simulink model "Complete_Model"  (R2023a)
settings: Solver=VariableStepAuto  StartTime=0.0  StopTime=22  MinStep=5e-5  RelTol=1e-3
values from neighbouring files (symbols this model uses):
  Cbus = 80e-3*n_wt_agg*u^2    (= 1.33333333333)    [param.m]
  D = 1e-3    (= 0.001)    [param.m]
  f = 50    [param.m]
  Fs = Vs * sqrt(2/3)/(2*pi*f)    [param.m]
  fsw = 4e3    (= 4000)    [param.m]
  Idr_rated = sqrt(2)*Is    [param.m]
  J = 127*n_wt_agg    (= 19050)    [param.m]
  ki_id = (wni^2)*Lr*sigma    (= 3.27733001332)    [param.m]
  ki_idg = (wnig^2)*Lg    (= 0.263189450696)    [param.m]
  ki_iq = ki_id    (= 3.27733001332)    [param.m]
  ki_iqg = ki_idg    (= 0.263189450696)    [param.m]
  ki_v = -300000*n_wt_agg    (= -45000000)    [param.m]
  Kopt = ((0.5*rho*pi*(Radius^5)*Cp_max)/(lambda_opt^3))    (= 296454.198186)    [param.m]
  kp_id = (2*wni*sigma*Lr) - Rr    (= 0.00384733333333)    [param.m]
  kp_idg = ((2*wnig*Lg)-Rg)    (= 0.00167538274858)    [param.m]
  kp_iq = kp_id    (= 0.00384733333333)    [param.m]
  kp_iqg = kp_idg    (= 0.00167538274858)    [param.m]
  kp_v = -1000*n_wt_agg    (= -150000)    [param.m]
  Kpg = 1/(1.5*Vr*sqrt(2/3))    [param.m]
  Kqg = -Kpg    [param.m]
  lambda_opt = 7.2    [param.m]
  Lg = 400e-6 /n_wt_agg    (= 2.66666666667e-06)    [param.m]
  Lm = 2.5e-3 /n_wt_agg    (= 1.66666666667e-05)    [param.m]
  Lr = Lm + Lsi    (= 1.72466666667e-05)    [param.m]
  Ls = Lm + Lsi    (= 1.72466666667e-05)    [param.m]
  Lsi = 0.087e-3 /n_wt_agg    (= 5.8e-07)    [param.m]
  N = 100    [param.m]
  n_wt_agg = 150    [param.m]
  p = 2    [param.m]
  Ps = 2e6*n_wt_agg    (= 300000000)    [param.m]
  Radius = 42    [param.m]
  Rg = 20e-6 /n_wt_agg    (= 1.33333333333e-07)    [param.m]
  rho = 1.225    [param.m]
  Rr = 2.9e-3 /n_wt_agg    (= 1.93333333333e-05)    [param.m]
  Rs = 2.6e-3 /n_wt_agg    (= 1.73333333333e-05)    [param.m]
  sigma = 1 - Lm^2 / (Ls*Lr)    (= 0.0661284179513)    [param.m]
  smax = 1/3    (= 0.333333333333)    [param.m]
  tab_lambda = [0.1:0.01:11.8]    [param.m]
  Vbus = 1150/u    (= 3450)    [param.m]
  Vs = 690    [param.m]
undefined symbols (not defined in the file or in its folder): Cp, D1, Delta, DiscIntNeverNeededParam, magnitude
libraries: spsSecondOrderFilterLib x133, spsThreePhaseVIMeasurementLib x58, spsPower3phInstantaneousLib x52, simulink x41, spsThreePhaseTransformerTwoWindingsLib x27, spsUniversalBridgeLib x22, spsPWMGenerator2LevelLib x22, spsSeriesRLCBranchLib x19, spsFirstOrderFilterLib x18, spsThreePhasePISectionLineLib x17, spsGroundLib x17, spsThreePhaseSeriesRLCBranchLib x17
(part 1 of 15)
settings Model: Creator=deleersnijde  LastModifiedBy=deleersnijde
settings DataIOCC: ReturnWorkspaceOutputs=off
settings RTWCC: GPUComputeCapability=5.0

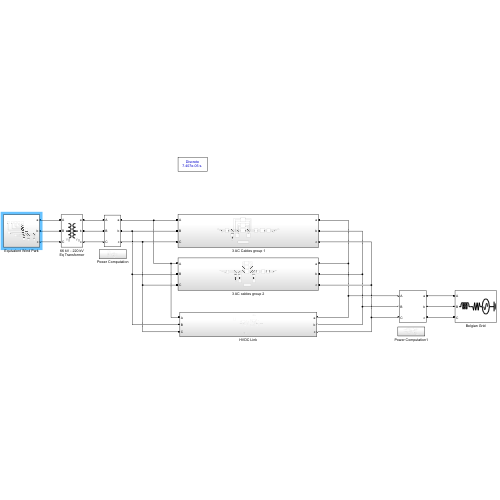
[thumbnail: Simulink's stored preview of the top-level diagram]
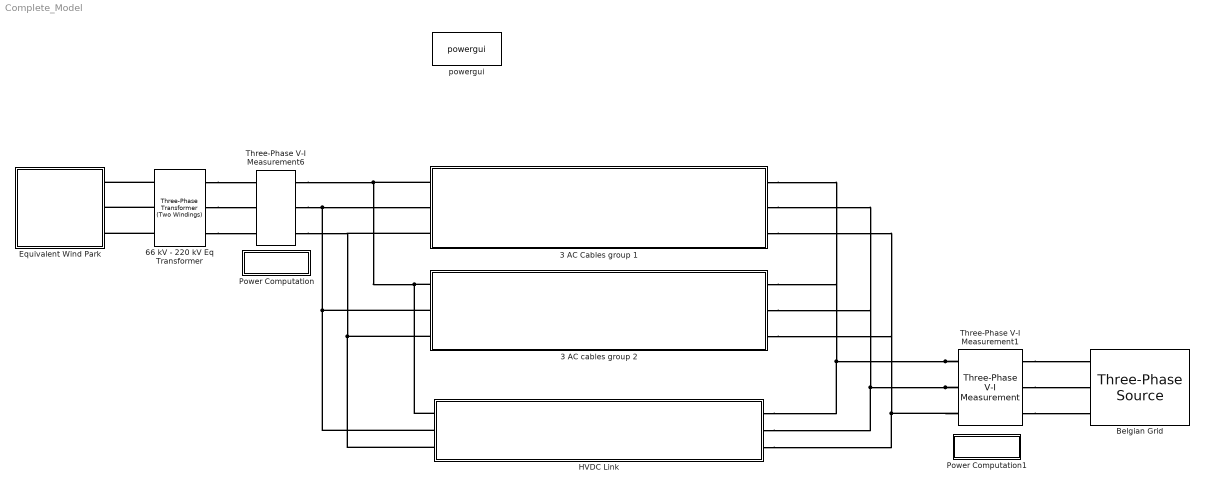
== system Complete_Model ==
  " 3 AC Cables group 1" : Subsystem
  3 AC cables group 2 : Subsystem
  66 kV - 220 kV Eq Transformer : spsThreePhaseTransformerTwoWindingsLib/Three-Phase Transformer (Two Windings)  Winding1Connection=Yg  Winding2Connection=Yg  CoreType=Three single-phase transformers  SetSaturation=off  Hysteresis=off  DataFile='hysteresis'  SetInitialFlux=off  Measurements=None  UNITS=pu  NominalPower=[ 3.5e9 , f ]  Winding1=[66e3 0.025/100 0.015]  Winding2=[220e3 0.025/100 0.015]  Rm=500  Lm=500  L0=0.5  Saturation=[0 0;0.22957 0.012546;95.654 0.015891]  InitialFluxes=[ 0.8 , -0.8 , 0.7 ]  BreakLoop=off  DiscreteSolver=Trapezoidal iterative  DataType=off  TransfoNumber=1
  Belgian Grid : spsThreePhaseSourceLib/Three-Phase Source  InternalConnection=Yg  VoltagePhases=off  Voltage=380e3  PhaseAngle=0  Voltage_phases=[25e3 25e3 25e3]/sqrt(3)  PhaseAngles_phases=[0 -120 +120]  Frequency=50  NonIdealSource=on  SpecifyImpedance=on  Resistance=0.8929  Inductance=16.58e-3  ShortCircuitLevel=1000000e6  BaseVoltage=380e3  XRratio=7  BusType=swing  Pref=10e3  Qref=0  Prefabc=[10e3, 10e3, 10e3]  Qrefabc=[0, 0, 0]  Qmin=-inf  Qmax=inf
  Equivalent Wind Park : Subsystem
  HVDC Link : Subsystem
  Power Computation : Subsystem
  Power Computation1 : Subsystem
  Three-Phase V-I Measurement1 : spsThreePhaseVIMeasurementLib/Three-Phase V-I Measurement  VoltageMeasurement=phase-to-ground  SetLabelV=on  LabelV=V_BE_grid_tot  Vpu=off  VpuLL=off  CurrentMeasurement=yes  SetLabelI=on  LabelI=I_BE_grid_tot  Ipu=off  Pbase=3*350e6  Vbase=66e3  OutputType=Complex  PhasorSimulation=off  PSBequivalent=0
  Three-Phase V-I Measurement6 : spsThreePhaseVIMeasurementLib/Three-Phase V-I Measurement  VoltageMeasurement=phase-to-ground  SetLabelV=on  LabelV=Vwf_tot  Vpu=off  VpuLL=off  CurrentMeasurement=yes  SetLabelI=on  LabelI=Iwf_tot  Ipu=off  Pbase=3*350e6  Vbase=66e3  OutputType=Complex  PhasorSimulation=off  PSBequivalent=0
  powergui : spspowerguiLib/powergui  Priority=1  SimulationMode=Discrete  SampleTime=7.4074e-05  frequency=60  Iterations=50  frequencyindice=50  Pbase=100e6  ErrMax=1e-4  UnitsV=kV  UnitsW=MW  buscounter=0  FunctionMessages=off  echomessages=off  EnableUseOfTLC=off  CurrentSourceSwitches=off  DisableSnubberDevices=off  DisableRonSwitches=off  DisableVfSwitches=off  DisplayEquations=off  AutomaticDiscreteSolvers=off  SolverType=Tustin/Backward Euler (TBE)  Interpol=off  ExternalGateDelay=off  methode=off  Ts=100  x0status=blocks  SPID=on  SwTol=0  HookPort=off  NonlinearTolerance=1e-4  nMaxIteration=100  ContinueOnMaxIteration=off  RestoreLinks=warning  ResistiveCurrentMeasurement=off  Frange=[0:2:500]  Ylog=off  Xlog=on  ShowGrid=off  save=off  variable=ZData  structure=0  ZoomFFT=off  StartTime=0.0  cycles=1  DisplayStyle=1  fundamental=60  FreqAxis=off  MaxFrequency=1000  frequencyindicesteady=1  RmsSteady=1  display=off
  connections:
  " 3 AC Cables group 1"/RConn3 -- 3 AC cables group 2/RConn3 -- HVDC Link/RConn3 -- Three-Phase V-I Measurement1/LConn3
  Three-Phase V-I Measurement1/LConn1 -- HVDC Link/RConn1 -- " 3 AC Cables group 1"/RConn1 -- 3 AC cables group 2/RConn1
  Three-Phase V-I Measurement1/LConn2 -- HVDC Link/RConn2 -- " 3 AC Cables group 1"/RConn2 -- 3 AC cables group 2/RConn2
  3 AC cables group 2/LConn2 -- HVDC Link/LConn2 -- " 3 AC Cables group 1"/LConn2 -- Three-Phase V-I Measurement6/RConn2
  3 AC cables group 2/LConn3 -- HVDC Link/LConn3 -- " 3 AC Cables group 1"/LConn3 -- Three-Phase V-I Measurement6/RConn3
  " 3 AC Cables group 1"/LConn1 -- Three-Phase V-I Measurement6/RConn1 -- HVDC Link/LConn1 -- 3 AC cables group 2/LConn1
  Three-Phase V-I Measurement1/RConn1 -- Belgian Grid/RConn1
  Three-Phase V-I Measurement1/RConn3 -- Belgian Grid/RConn3
  Three-Phase V-I Measurement1/RConn2 -- Belgian Grid/RConn2
  66 kV - 220 kV Eq Transformer/RConn1 -- Three-Phase V-I Measurement6/LConn1
  66 kV - 220 kV Eq Transformer/RConn2 -- Three-Phase V-I Measurement6/LConn2
  66 kV - 220 kV Eq Transformer/RConn3 -- Three-Phase V-I Measurement6/LConn3
  66 kV - 220 kV Eq Transformer/LConn1 -- Equivalent Wind Park/RConn1
  66 kV - 220 kV Eq Transformer/LConn2 -- Equivalent Wind Park/RConn2
  66 kV - 220 kV Eq Transformer/LConn3 -- Equivalent Wind Park/RConn3

== system Complete_Model/ 3 AC Cables group 1 ==
  220kV - 380kV : spsThreePhaseTransformerTwoWindingsLib/Three-Phase Transformer (Two Windings)  Winding1Connection=Yg  Winding2Connection=Yg  CoreType=Three single-phase transformers  SetSaturation=off  Hysteresis=off  DataFile='hysteresis'  SetInitialFlux=off  Measurements=None  UNITS=pu  NominalPower=[ 3*350e6 , 50 ]  Winding1=[ 220e3 , 0.002 , 0.08 ]  Winding2=[ 380e3 , 0.002 , 0.08 ]  Rm=500  Lm=500  L0=0.5  Saturation=[ 0,0 ; 0.0024,1.2 ; 1.0,1.52 ]  InitialFluxes=[ 0.8 , -0.8 , 0.7 ]  BreakLoop=off  DiscreteSolver=Trapezoidal iterative  DataType=off  TransfoNumber=1
  220kV export cable1 : spsThreePhasePISectionLineLib/Three-Phase PI Section Line  LConnTagsString=a|b|c  RConnTagsString=A|B|C  Length=45  Frequency=50  Resistances=[ 0.048 0.048]  Inductances="[ 0.37e-3  0.37e-3]"  Capacitances=[0.18e-6 0.18e-6]
  220kV export cable2 : spsThreePhasePISectionLineLib/Three-Phase PI Section Line  LConnTagsString=a|b|c  RConnTagsString=A|B|C  Length=45  Frequency=50  Resistances=[ 0.048 0.048]  Inductances="[ 0.37e-3  0.37e-3]"  Capacitances=[0.18e-6 0.18e-6]
  220kV export cable3 : spsThreePhasePISectionLineLib/Three-Phase PI Section Line  LConnTagsString=a|b|c  RConnTagsString=A|B|C  Length=45  Frequency=50  Resistances=[ 0.048 0.048]  Inductances="[ 0.37e-3  0.37e-3]"  Capacitances=[0.18e-6 0.18e-6]
  Ground11 : spsGroundLib/Ground  LConnTagsString=a
  Power Computations : Subsystem
  Shunt Reactor 1 : spsThreePhaseSeriesRLCLoadLib/Three-Phase Series RLC Load  LConnTagsString=A|B|C  Configuration=Y (grounded)  NominalVoltage=220e3  Vabc=[1e3 1e3 1e3]  Vabcp=[1e3 1e3 1e3]  NominalFrequency=50  UnbalancedPower=off  ActivePower=0  InductivePower=175e6  CapacitivePower=0  Pabc=[10e3 9e3 11e3]  QLabc=[100 90 110]  QCabc=[100 90 110]  Pabcp=[10e3 9e3 11e3]  QLabcp=[100 90 110]  QCabcp=[100 90 110]  Measurements=None  LoadType=constant Z
  Shunt Reactor 2 : spsThreePhaseSeriesRLCLoadLib/Three-Phase Series RLC Load  LConnTagsString=A|B|C  Configuration=Y (grounded)  NominalVoltage=220e3  Vabc=[1e3 1e3 1e3]  Vabcp=[1e3 1e3 1e3]  NominalFrequency=50  UnbalancedPower=off  ActivePower=0  InductivePower=175e6  CapacitivePower=0  Pabc=[10e3 9e3 11e3]  QLabc=[100 90 110]  QCabc=[100 90 110]  Pabcp=[10e3 9e3 11e3]  QLabcp=[100 90 110]  QCabcp=[100 90 110]  Measurements=None  LoadType=constant Z
  Snubber Shunt5 : spsThreePhaseSeriesRLCBranchLib/Three-Phase Series RLC Branch  LConnTagsString=A|B|C  RConnTagsString=A|B|C  BranchType=R  Resistance=10e6  Inductance=Lg  Capacitance=1e-6  Measurements=None
  Three-Phase V-I Measurement3 : spsThreePhaseVIMeasurementLib/Three-Phase V-I Measurement  VoltageMeasurement=phase-to-ground  SetLabelV=on  LabelV=Vcontinent220  Vpu=off  VpuLL=off  CurrentMeasurement=yes  SetLabelI=on  LabelI=Icontinent220  Ipu=off  Pbase=3*350e6  Vbase=220e3  OutputType=Complex  PhasorSimulation=off  PSBequivalent=0
  Three-Phase V-I Measurement6 : spsThreePhaseVIMeasurementLib/Three-Phase V-I Measurement  VoltageMeasurement=phase-to-ground  SetLabelV=on  LabelV=Ven_isl  Vpu=off  VpuLL=off  CurrentMeasurement=yes  SetLabelI=on  LabelI=Ien_isl  Ipu=off  Pbase=3*350e6  Vbase=66e3  OutputType=Complex  PhasorSimulation=off  PSBequivalent=0
  Three-Phase V-I Measurement8 : spsThreePhaseVIMeasurementLib/Three-Phase V-I Measurement  VoltageMeasurement=phase-to-ground  SetLabelV=on  LabelV=Vcontinent  Vpu=off  VpuLL=off  CurrentMeasurement=yes  SetLabelI=on  LabelI=Icontinent  Ipu=off  Pbase=3*350e6  Vbase=380e3  OutputType=Complex  PhasorSimulation=off  PSBequivalent=0
  a : PMIOPort  Side=Right
  b : PMIOPort  Port=2  Side=Right
  c : PMIOPort  Port=3  Side=Right
  A : PMIOPort  Port=4  Side=Left
  B : PMIOPort  Port=5  Side=Left
  C : PMIOPort  Port=6  Side=Left
  connections:
  Three-Phase V-I Measurement6/RConn2 -- 220kV export cable2/LConn2 -- 220kV export cable3/LConn2 -- Shunt Reactor 1/LConn2 -- 220kV export cable1/LConn2 -- Snubber Shunt5/LConn2
  Shunt Reactor 2/LConn3 -- Three-Phase V-I Measurement3/LConn3 -- 220kV export cable2/RConn3 -- 220kV export cable3/RConn3 -- 220kV export cable1/RConn3
  Snubber Shunt5/RConn2 -- Snubber Shunt5/RConn3 -- Ground11/LConn1 -- Snubber Shunt5/RConn1
  Shunt Reactor 2/LConn1 -- 220kV export cable3/RConn1 -- 220kV export cable1/RConn1 -- Three-Phase V-I Measurement3/LConn1 -- 220kV export cable2/RConn1
  Three-Phase V-I Measurement8/LConn2 -- 220kV - 380kV/RConn2
  Snubber Shunt5/LConn3 -- Shunt Reactor 1/LConn3 -- 220kV export cable2/LConn3 -- 220kV export cable3/LConn3 -- 220kV export cable1/LConn3 -- Three-Phase V-I Measurement6/RConn3
  Shunt Reactor 2/LConn2 -- 220kV export cable2/RConn2 -- 220kV export cable3/RConn2 -- Three-Phase V-I Measurement3/LConn2 -- 220kV export cable1/RConn2
  Three-Phase V-I Measurement3/RConn1 -- 220kV - 380kV/LConn1
  Three-Phase V-I Measurement6/RConn1 -- Shunt Reactor 1/LConn1 -- 220kV export cable2/LConn1 -- 220kV export cable3/LConn1 -- 220kV export cable1/LConn1 -- Snubber Shunt5/LConn1
  Three-Phase V-I Measurement3/RConn2 -- 220kV - 380kV/LConn2
  Three-Phase V-I Measurement8/LConn1 -- 220kV - 380kV/RConn1
  Three-Phase V-I Measurement3/RConn3 -- 220kV - 380kV/LConn3
  Three-Phase V-I Measurement8/LConn3 -- 220kV - 380kV/RConn3
  Three-Phase V-I Measurement8/RConn1 -- a/RConn1
  Three-Phase V-I Measurement8/RConn3 -- c/RConn1
  Three-Phase V-I Measurement8/RConn2 -- b/RConn1
  B/RConn1 -- Three-Phase V-I Measurement6/LConn2
  C/RConn1 -- Three-Phase V-I Measurement6/LConn3
  A/RConn1 -- Three-Phase V-I Measurement6/LConn1

== system Complete_Model/3 AC cables group 2 ==
  220kV - 380kV1 : spsThreePhaseTransformerTwoWindingsLib/Three-Phase Transformer (Two Windings)  Winding1Connection=Yg  Winding2Connection=Yg  CoreType=Three single-phase transformers  SetSaturation=off  Hysteresis=off  DataFile='hysteresis'  SetInitialFlux=off  Measurements=None  UNITS=pu  NominalPower=[ 3*350e6 , 50 ]  Winding1=[ 220e3 , 0.002 , 0.08 ]  Winding2=[ 380e3 , 0.002 , 0.08 ]  Rm=500  Lm=500  L0=0.5  Saturation=[ 0,0 ; 0.0024,1.2 ; 1.0,1.52 ]  InitialFluxes=[ 0.8 , -0.8 , 0.7 ]  BreakLoop=off  DiscreteSolver=Trapezoidal iterative  DataType=off  TransfoNumber=1
  220kV export cable4 : spsThreePhasePISectionLineLib/Three-Phase PI Section Line  LConnTagsString=a|b|c  RConnTagsString=A|B|C  Length=45  Frequency=50  Resistances=[ 0.048 0.048]  Inductances="[ 0.37e-3  0.37e-3]"  Capacitances=[0.18e-6 0.18e-6]
  220kV export cable5 : spsThreePhasePISectionLineLib/Three-Phase PI Section Line  LConnTagsString=a|b|c  RConnTagsString=A|B|C  Length=45  Frequency=50  Resistances=[ 0.048 0.048]  Inductances="[ 0.37e-3  0.37e-3]"  Capacitances=[0.18e-6 0.18e-6]
  220kV export cable6 : spsThreePhasePISectionLineLib/Three-Phase PI Section Line  LConnTagsString=a|b|c  RConnTagsString=A|B|C  Length=45  Frequency=50  Resistances=[ 0.048 0.048]  Inductances="[ 0.37e-3  0.37e-3]"  Capacitances=[0.18e-6 0.18e-6]
  Ground3 : spsGroundLib/Ground  LConnTagsString=a
  Power Computations : Subsystem
  Snubber Shunt4 : spsThreePhaseSeriesRLCBranchLib/Three-Phase Series RLC Branch  LConnTagsString=A|B|C  RConnTagsString=A|B|C  BranchType=R  Resistance=10e6  Inductance=Lg  Capacitance=1e-6  Measurements=None
  Three-Phase Series RLC Load2 : spsThreePhaseSeriesRLCLoadLib/Three-Phase Series RLC Load  LConnTagsString=A|B|C  Configuration=Y (grounded)  NominalVoltage=220e3  Vabc=[1e3 1e3 1e3]  Vabcp=[1e3 1e3 1e3]  NominalFrequency=50  UnbalancedPower=off  ActivePower=0  InductivePower=175e6  CapacitivePower=0  Pabc=[10e3 9e3 11e3]  QLabc=[100 90 110]  QCabc=[100 90 110]  Pabcp=[10e3 9e3 11e3]  QLabcp=[100 90 110]  QCabcp=[100 90 110]  Measurements=None  LoadType=constant Z
  Three-Phase Series RLC Load3 : spsThreePhaseSeriesRLCLoadLib/Three-Phase Series RLC Load  LConnTagsString=A|B|C  Configuration=Y (grounded)  NominalVoltage=220e3  Vabc=[1e3 1e3 1e3]  Vabcp=[1e3 1e3 1e3]  NominalFrequency=50  UnbalancedPower=off  ActivePower=0  InductivePower=175e6  CapacitivePower=0  Pabc=[10e3 9e3 11e3]  QLabc=[100 90 110]  QCabc=[100 90 110]  Pabcp=[10e3 9e3 11e3]  QLabcp=[100 90 110]  QCabcp=[100 90 110]  Measurements=None  LoadType=constant Z
  Three-Phase V-I Measurement1 : spsThreePhaseVIMeasurementLib/Three-Phase V-I Measurement  VoltageMeasurement=phase-to-ground  SetLabelV=on  LabelV=Vsh_wind2  Vpu=off  VpuLL=off  CurrentMeasurement=yes  SetLabelI=on  LabelI=Ish_wind2  Ipu=off  Pbase=3*350e6  Vbase=66e3  OutputType=Complex  PhasorSimulation=off  PSBequivalent=0
  Three-Phase V-I Measurement10 : spsThreePhaseVIMeasurementLib/Three-Phase V-I Measurement  VoltageMeasurement=phase-to-ground  SetLabelV=on  LabelV=Ven_isl2  Vpu=off  VpuLL=off  CurrentMeasurement=yes  SetLabelI=on  LabelI=Ien_isl2  Ipu=off  Pbase=3*350e6  Vbase=66e3  OutputType=Complex  PhasorSimulation=off  PSBequivalent=0
  Three-Phase V-I Measurement11 : spsThreePhaseVIMeasurementLib/Three-Phase V-I Measurement  VoltageMeasurement=phase-to-ground  SetLabelV=on  LabelV=Vcontinent2  Vpu=off  VpuLL=off  CurrentMeasurement=yes  SetLabelI=on  LabelI=Icontinent2  Ipu=off  Pbase=3*350e6  Vbase=380e3  OutputType=Complex  PhasorSimulation=off  PSBequivalent=0
  Three-Phase V-I Measurement2 : spsThreePhaseVIMeasurementLib/Three-Phase V-I Measurement  VoltageMeasurement=phase-to-ground  SetLabelV=on  LabelV=Vsh_grid2  Vpu=off  VpuLL=off  CurrentMeasurement=yes  SetLabelI=on  LabelI=Ish_grid2  Ipu=off  Pbase=3*350e6  Vbase=66e3  OutputType=Complex  PhasorSimulation=off  PSBequivalent=0
  Three-Phase V-I Measurement9 : spsThreePhaseVIMeasurementLib/Three-Phase V-I Measurement  VoltageMeasurement=phase-to-ground  SetLabelV=on  LabelV=Vcontinent220_2  Vpu=off  VpuLL=off  CurrentMeasurement=yes  SetLabelI=on  LabelI=Icontinent220_2  Ipu=off  Pbase=3*350e6  Vbase=220e3  OutputType=Complex  PhasorSimulation=off  PSBequivalent=0
  a : PMIOPort  Side=Right
  b : PMIOPort  Port=2  Side=Right
  c : PMIOPort  Port=3  Side=Right
  A : PMIOPort  Port=4  Side=Left
  B : PMIOPort  Port=5  Side=Left
  C : PMIOPort  Port=6  Side=Left
  connections:
  Three-Phase V-I Measurement9/RConn2 -- 220kV - 380kV1/LConn2
  Three-Phase V-I Measurement9/LConn2 -- 220kV export cable6/RConn2 -- 220kV export cable4/RConn2 -- 220kV export cable5/RConn2 -- Three-Phase V-I Measurement2/LConn2
  220kV export cable6/RConn1 -- Three-Phase V-I Measurement2/LConn1 -- Three-Phase V-I Measurement9/LConn1 -- 220kV export cable4/RConn1 -- 220kV export cable5/RConn1
  220kV export cable6/RConn3 -- Three-Phase V-I Measurement2/LConn3 -- Three-Phase V-I Measurement9/LConn3 -- 220kV export cable5/RConn3 -- 220kV export cable4/RConn3
  Three-Phase V-I Measurement11/LConn2 -- 220kV - 380kV1/RConn2
  Three-Phase V-I Measurement11/RConn3 -- c/RConn1
  Three-Phase V-I Measurement10/LConn1 -- A/RConn1
  Three-Phase V-I Measurement10/RConn3 -- Snubber Shunt4/LConn3 -- Three-Phase V-I Measurement1/LConn3 -- 220kV export cable6/LConn3 -- 220kV export cable5/LConn3 -- 220kV export cable4/LConn3
  Three-Phase V-I Measurement11/LConn3 -- 220kV - 380kV1/RConn3
  220kV export cable6/LConn2 -- Three-Phase V-I Measurement1/LConn2 -- Three-Phase V-I Measurement10/RConn2 -- Snubber Shunt4/LConn2 -- 220kV export cable5/LConn2 -- 220kV export cable4/LConn2
  220kV export cable6/LConn1 -- Three-Phase V-I Measurement1/LConn1 -- Three-Phase V-I Measurement10/RConn1 -- Snubber Shunt4/LConn1 -- 220kV export cable4/LConn1 -- 220kV export cable5/LConn1
  Ground3/LConn1 -- Snubber Shunt4/RConn1 -- Snubber Shunt4/RConn3 -- Snubber Shunt4/RConn2
  Three-Phase V-I Measurement11/RConn2 -- b/RConn1
  Three-Phase V-I Measurement9/RConn3 -- 220kV - 380kV1/LConn3
  Three-Phase V-I Measurement10/LConn2 -- B/RConn1
  Three-Phase V-I Measurement11/LConn1 -- 220kV - 380kV1/RConn1
  Three-Phase V-I Measurement9/RConn1 -- 220kV - 380kV1/LConn1
  Three-Phase V-I Measurement11/RConn1 -- a/RConn1
  Three-Phase V-I Measurement10/LConn3 -- C/RConn1
  Three-Phase Series RLC Load2/LConn3 -- Three-Phase V-I Measurement1/RConn3
  Three-Phase Series RLC Load2/LConn2 -- Three-Phase V-I Measurement1/RConn2
  Three-Phase Series RLC Load2/LConn1 -- Three-Phase V-I Measurement1/RConn1
  Three-Phase V-I Measurement2/RConn1 -- Three-Phase Series RLC Load3/LConn1
  Three-Phase V-I Measurement2/RConn3 -- Three-Phase Series RLC Load3/LConn3
  Three-Phase Series RLC Load3/LConn2 -- Three-Phase V-I Measurement2/RConn2

== system Complete_Model/Equivalent Wind Park ==
  300MW Eq WT1 : Subsystem
  300MW Eq WT10 : Subsystem
  300MW Eq WT11 : Subsystem
  300MW Eq WT2 : Subsystem
  300MW Eq WT3 : Subsystem
  300MW Eq WT4 : Subsystem
  300MW Eq WT5 : Subsystem
  300MW Eq WT6 : Subsystem
  300MW Eq WT7 : Subsystem
  300MW Eq WT8 : Subsystem
  300MW Eq WT9 : Subsystem
  a : PMIOPort  Side=Right
  b : PMIOPort  Port=2  Side=Right
  c : PMIOPort  Port=3  Side=Right
  connections:
  300MW Eq WT1/LConn3 -- 300MW Eq WT3/LConn3 -- 300MW Eq WT4/LConn3 -- 300MW Eq WT5/LConn3 -- 300MW Eq WT6/LConn3 -- c/RConn1 -- 300MW Eq WT11/LConn3 -- 300MW Eq WT10/LConn3 -- 300MW Eq WT8/LConn3 -- 300MW Eq WT9/LConn3 -- 300MW Eq WT7/LConn3 -- 300MW Eq WT2/LConn3
  300MW Eq WT1/LConn1 -- 300MW Eq WT3/LConn1 -- 300MW Eq WT4/LConn1 -- 300MW Eq WT5/LConn1 -- 300MW Eq WT6/LConn1 -- 300MW Eq WT9/LConn1 -- 300MW Eq WT8/LConn1 -- a/RConn1 -- 300MW Eq WT11/LConn1 -- 300MW Eq WT10/LConn1 -- 300MW Eq WT7/LConn1 -- 300MW Eq WT2/LConn1
  300MW Eq WT1/LConn2 -- 300MW Eq WT3/LConn2 -- 300MW Eq WT4/LConn2 -- 300MW Eq WT5/LConn2 -- 300MW Eq WT6/LConn2 -- b/RConn1 -- 300MW Eq WT11/LConn2 -- 300MW Eq WT10/LConn2 -- 300MW Eq WT8/LConn2 -- 300MW Eq WT9/LConn2 -- 300MW Eq WT7/LConn2 -- 300MW Eq WT2/LConn2

== system Complete_Model/HVDC Link ==
  220 kV - 525 kV L = 0.1947 pu : spsThreePhaseTransformerTwoWindingsLib/Three-Phase Transformer (Two Windings)  Winding1Connection=Yg  Winding2Connection=Yg  CoreType=Three single-phase transformers  SetSaturation=off  Hysteresis=off  DataFile='hysteresis'  SetInitialFlux=off  Measurements=None  UNITS=pu  NominalPower=[ 2e9 , 50 ]  Winding1=[2.2e+05 0.0025 0.1947/2]  Winding2=[525e3 0.0025 0.1947/2]  Rm=500  Lm=500  L0=0.5  Saturation=[0 0;0.0003 1.2;0.125 1.52]  InitialFluxes=[ 0.8 , -0.8 , 0.7 ]  BreakLoop=off  DiscreteSolver=Trapezoidal iterative  DataType=off  TransfoNumber=1
  45-km cable neg dc : spsPiSectionLineLib/Pi Section Line  LConnTagsString=__new0  RConnTagsString=__new0  Phases=1  PiSections=1  Length=45  Frequency=50  Resistance=0.0072  Inductance=1.5e-004  Capacitance=0.22e-06  SpecifyConductance=off  Conductance=0  Measurements=None  Measurements2=None
  45-km cable pos dc : spsPiSectionLineLib/Pi Section Line  LConnTagsString=__new0  RConnTagsString=__new0  Phases=1  PiSections=1  Length=45  Frequency=50  Resistance=0.0072  Inductance=1.5e-004  Capacitance=0.22e-06  SpecifyConductance=off  Conductance=0  Measurements=None  Measurements2=None
  525 kV - 380 kV L = 0.1947 pu : spsThreePhaseTransformerTwoWindingsLib/Three-Phase Transformer (Two Windings)  Winding1Connection=Yg  Winding2Connection=Yg  CoreType=Three single-phase transformers  SetSaturation=off  Hysteresis=off  DataFile='hysteresis'  SetInitialFlux=off  Measurements=None  UNITS=pu  NominalPower=[ 2e9 , 50 ]  Winding1=[380e3 0.0025 0.1947/2]  Winding2=[525e3 0.0025 0.1947/2]  Rm=500  Lm=500  L0=0.5  Saturation=[0 0;0.0003 1.2;0.125 1.52]  InitialFluxes=[ 0.8 , -0.8 , 0.7 ]  BreakLoop=off  DiscreteSolver=Trapezoidal iterative  DataType=off  TransfoNumber=1
  AC Filter gride side : spsThreePhaseSeriesRLCBranchLib/Three-Phase Series RLC Branch  LConnTagsString=A|B|C  RConnTagsString=A|B|C  BranchType=RC  Resistance=62.64  Inductance=2.9042e-3  Capacitance=1.1549e-6  Measurements=None
  AC Filter wfside : spsThreePhaseSeriesRLCBranchLib/Three-Phase Series RLC Branch  LConnTagsString=A|B|C  RConnTagsString=A|B|C  BranchType=RC  Resistance=62.64  Inductance=2.9042e-3  Capacitance=1.1549e-6  Measurements=None
  Block2 : Step  Time=0.1  Before=1  After=0  SampleTime=7.4074e-05
  Block3 : Step  Time=0.3  Before=1  After=0  SampleTime=7.4074e-05
  Constant1 : Constant
  Constant10 : Constant
  Constant11 : Constant  Value=0
  Constant12 : Constant  Value=0
  Current Measurement1 : spsCurrentMeasurementLib/Current Measurement  PhasorSimulation=off  OutputType=Complex  PSBequivalent=0
  Current Measurement2 : spsCurrentMeasurementLib/Current Measurement  PhasorSimulation=off  OutputType=Complex  PSBequivalent=0
  DC Capacitor : spsSeriesRLCBranchLib/Series RLC Branch  LConnTagsString=__new0  RConnTagsString=__new0  BranchType=C  Resistance=1  Inductance=1e-3  SetiL0=off  InitialCurrent=0  Capacitance=25.4e-6  Setx0=off  InitialVoltage=525e5  Measurements=None
  Demux1 : Demux  Outputs=3
  Grid side control : Subsystem
  Ground{1..2,4,6} (4 alike) : spsGroundLib/Ground  LConnTagsString=a
  Lp2 : spsSeriesRLCBranchLib/Series RLC Branch  LConnTagsString=__new0  RConnTagsString=__new0  BranchType=RL  Resistance=0.0692  Inductance=0.02205  SetiL0=off  InitialCurrent=0  Capacitance=[]  Setx0=off  InitialVoltage=0  Measurements=Branch current
  Lp3 : spsSeriesRLCBranchLib/Series RLC Branch  LConnTagsString=__new0  RConnTagsString=__new0  BranchType=RL  Resistance=0.0692  Inductance=0.02205  SetiL0=off  InitialCurrent=0  Capacitance=[]  Setx0=off  InitialVoltage=0  Measurements=Branch current
  Lp4 : spsSeriesRLCBranchLib/Series RLC Branch  LConnTagsString=__new0  RConnTagsString=__new0  BranchType=RL  Resistance=0.0692  Inductance=0.02205  SetiL0=off  InitialCurrent=0  Capacitance=[]  Setx0=off  InitialVoltage=0  Measurements=Branch current
  PWM Generator (3-Level)2 : spsPWMGenerator3LevelLib/PWM Generator (3-Level)  ModulatorType=Three-phase bridge (12 pulses)  ModulatorMode=Unsynchronized  nF=27  Fc=27*50  ModulatingSignals=off  m=0.8  Freq=60  Phase=0  Ts=7.4074e-05
  PWM Generator (3-Level)3 : spsPWMGenerator3LevelLib/PWM Generator (3-Level)  ModulatorType=Three-phase bridge (12 pulses)  ModulatorMode=Unsynchronized  nF=27  Fc=27*50  ModulatingSignals=off  m=0.8  Freq=60  Phase=0  Ts=7.4074e-05
  Phase Reactor grid L = 0.0781 pu : spsThreePhaseSeriesRLCBranchLib/Three-Phase Series RLC Branch  LConnTagsString=A|B|C  RConnTagsString=A|B|C  BranchType=RL  Resistance=(0.015/100)*(525^2 / 2000)  Inductance=0.0781  Capacitance=1e-6  Measurements=None
  Phase Reactor wfside L = 0.0781 pu : spsThreePhaseSeriesRLCBranchLib/Three-Phase Series RLC Branch  LConnTagsString=A|B|C  RConnTagsString=A|B|C  BranchType=RL  Resistance=(0.015/100)*(525^2 / 2000)  Inductance=0.0781  Capacitance=1e-6  Measurements=None
  Power compuations Grid Side : Subsystem
  Power compuations Wind Farm Side : Subsystem
  Power losses & DC power : Subsystem
  Series RLC Branch4 : spsSeriesRLCBranchLib/Series RLC Branch  LConnTagsString=__new0  RConnTagsString=__new0  BranchType=C  Resistance=1  Inductance=1e-3  SetiL0=off  InitialCurrent=0  Capacitance=25.4e-6  Setx0=off  InitialVoltage=525e5  Measurements=None
  Series RLC Branch5 : spsSeriesRLCBranchLib/Series RLC Branch  LConnTagsString=__new0  RConnTagsString=__new0  BranchType=C  Resistance=1  Inductance=1e-3  SetiL0=off  InitialCurrent=0  Capacitance=25.4e-6  Setx0=off  InitialVoltage=525e5  Measurements=None
  Series RLC Branch6 : spsSeriesRLCBranchLib/Series RLC Branch  LConnTagsString=__new0  RConnTagsString=__new0  BranchType=C  Resistance=1  Inductance=1e-3  SetiL0=off  InitialCurrent=0  Capacitance=25.4e-6  Setx0=off  InitialVoltage=525e5  Measurements=None
  Smoothing Reactor : spsSeriesRLCBranchLib/Series RLC Branch  LConnTagsString=__new0  RConnTagsString=__new0  BranchType=RL  Resistance=0.0692  Inductance=0.02205  SetiL0=off  InitialCurrent=0  Capacitance=[]  Setx0=off  InitialVoltage=0  Measurements=Branch current
  Step3 : Step  Time=0.05  Before=1  After=-1  SampleTime=0
  Subsystem : Subsystem
  Subsystem1 : Subsystem
  Subsystem7 : Subsystem
  Switch3 : Switch  Criteria=u2 > Threshold  InputSameDT=off
  Switch4 : Switch  Criteria=u2 > Threshold  InputSameDT=off
  Switch5 : Switch  Criteria=u2 > Threshold  SaturateOnIntegerOverflow=off  InputSameDT=off
  Three-Level Bridge2 : spsThreeLevelBridgeLib/Three-Level Bridge  Arms=3  SnubberResistance=5000  SbubberCapacitance=1e-6  Device=IGBT / Diodes  Ron=1e-3  ForwardVoltages="[  0  0  ]"  Measurements=None
      settings: LibrarySourceBlock=sps_lib/Power Electronics/Three-Level Bridge
  Three-Level Bridge3 : spsThreeLevelBridgeLib/Three-Level Bridge  Arms=3  SnubberResistance=5000  SbubberCapacitance=1e-6  Device=IGBT / Diodes  Ron=1e-3  ForwardVoltages="[  0  0  ]"  Measurements=None
      settings: LibrarySourceBlock=sps_lib/Power Electronics/Three-Level Bridge
  Three-Phase V-I Measurement1 : spsThreePhaseVIMeasurementLib/Three-Phase V-I Measurement  VoltageMeasurement=phase-to-ground  SetLabelV=on  LabelV=Eabc_windfarm  Vpu=on  VpuLL=off  CurrentMeasurement=yes  SetLabelI=on  LabelI=Iabc_windfarm  Ipu=on  Pbase=2e9  Vbase=220e3  OutputType=Complex  PhasorSimulation=off  PSBequivalent=0
  Three-Phase V-I Measurement4 : spsThreePhaseVIMeasurementLib/Three-Phase V-I Measurement  VoltageMeasurement=phase-to-ground  SetLabelV=on  LabelV=Eabc_grid  Vpu=on  VpuLL=off  CurrentMeasurement=yes  SetLabelI=on  LabelI=Iabc_grid  Ipu=on  Pbase=2e9  Vbase=380e3  OutputType=Complex  PhasorSimulation=off  PSBequivalent=0
  Three-Phase V-I Measurement5 : spsThreePhaseVIMeasurementLib/Three-Phase V-I Measurement  VoltageMeasurement=phase-to-ground  SetLabelV=on  LabelV=Uconv_grid  Vpu=on  VpuLL=off  CurrentMeasurement=no  SetLabelI=on  LabelI=Iabc_grid  Ipu=on  Pbase=2e9  Vbase=525e3  OutputType=Complex  PhasorSimulation=off  PSBequivalent=0
  Three-Phase V-I Measurement7 : spsThreePhaseVIMeasurementLib/Three-Phase V-I Measurement  VoltageMeasurement=phase-to-ground  SetLabelV=on  LabelV=Uconv_windfarm  Vpu=on  VpuLL=off  CurrentMeasurement=yes  SetLabelI=on  LabelI=Iconv_windfarm  Ipu=on  Pbase=2e9  Vbase=525e3  OutputType=Complex  PhasorSimulation=off  PSBequivalent=0
  To Workspace1 : ToWorkspace  VariableName=simout1  SaveFormat=Structure With Time  SampleTime=-1
  To Workspace3 : ToWorkspace  VariableName=Vdc_ref  SaveFormat=Timeseries  SampleTime=-1
  ToFile_Vdc_ref : ToFile  Filename=Vdc_ref.mat  SaveFormat=Timeseries
  ToFile_simout1 : ToFile  Filename=simout1.mat  SaveFormat=Timeseries
  V_DC Measure : Subsystem
  V_DC_neg Measure : Subsystem
  Voltage Measurement5 : spsVoltageMeasurementLib/Voltage Measurement  PhasorSimulation=off  OutputType=Complex  PSBequivalent=0
  Wind farm side control : Subsystem
  big scope : Subsystem
  powergui : spspowerguiLib/powergui  Priority=1  SimulationMode=Discrete  SampleTime=7.4074e-05  frequency=60  Iterations=50  frequencyindice=50  Pbase=100e6  ErrMax=1e-4  UnitsV=kV  UnitsW=MW  buscounter=0  FunctionMessages=off  echomessages=off  EnableUseOfTLC=off  CurrentSourceSwitches=off  DisableSnubberDevices=off  DisableRonSwitches=off  DisableVfSwitches=off  DisplayEquations=off  AutomaticDiscreteSolvers=off  SolverType=Tustin/Backward Euler (TBE)  Interpol=off  ExternalGateDelay=off  methode=off  Ts=100  x0status=blocks  SPID=on  SwTol=0  HookPort=off  NonlinearTolerance=1e-4  nMaxIteration=100  ContinueOnMaxIteration=off  RestoreLinks=warning  ResistiveCurrentMeasurement=off  Frange=[0:2:500]  Ylog=off  Xlog=on  ShowGrid=off  save=off  variable=ZData  structure=0  ZoomFFT=off  StartTime=0.0  cycles=1  DisplayStyle=1  fundamental=60  FreqAxis=off  MaxFrequency=1000  frequencyindicesteady=1  RmsSteady=1  display=off
  A : PMIOPort  Side=Left
  B : PMIOPort  Port=2  Side=Left
  C : PMIOPort  Port=3  Side=Left
  a : PMIOPort  Port=4  Side=Right
  b : PMIOPort  Port=5  Side=Right
  c : PMIOPort  Port=6  Side=Right
  tag scope: I_DC=global, I_DC_neg=global, Va_conv_windfarm=global, Vb_conv_windfarm=global, Vc_conv_windfarm=global, Vdc_ref=global
  wires:
  Subsystem1/1(Qref_grid) -> Grid side control/4(Qref_grid)
  Subsystem7/1(Pref_hvdc) -> Wind farm side control/2(Pref_windfarm)
  Current Measurement1/1 -> [I_DC]
  Current Measurement2/1 -> [I_DC_neg]
  Grid side control/1(Vref_abc) -> PWM Generator (3-Level)2/1
  [pulses_windfarm] -> Three-Level Bridge3/1
  Step3/1 -> Switch5/2
  [Vdc_ref] -> Switch5/3
  [Qmes_hvdc_BE] -> Grid side control/3(Q_grid)
  Switch5/1 -> To Workspace3/1, ToFile_Vdc_ref/1  "Vdc_ref"
  [Q_hvdc_wfside] -> Wind farm side control/3(Q_windfarm)
  PWM Generator (3-Level)2/1 -> Switch3/3
  Constant10/1 -> Switch5/1
  [Vdcp_mes] -> Grid side control/1(Vdc_mes)
  Switch3/1 -> [pulses_grid]
  [pulses_grid] -> Three-Level Bridge2/1
  Constant1/1 -> [Vdc_ref], Grid side control/2(Vdc_ref)
  PWM Generator (3-Level)3/1 -> Switch4/3
  Wind farm side control/1(Vref_abc) -> PWM Generator (3-Level)3/1
  [Uconv_windfarm] -> Demux1/1
  Constant12/1 -> Switch4/1
  Demux1/3 -> [Vc_conv_windfarm]
  Demux1/1 -> ToFile_simout1/1, To Workspace1/1, [Va_conv_windfarm]  "simout1"
  Demux1/2 -> [Vb_conv_windfarm]
  Switch4/1 -> [pulses_windfarm]
  Constant11/1 -> Switch3/1
  Block3/1 -> Switch4/2
  [P_hvdc_wfside] -> Wind farm side control/1(P_windfarm)
  Block2/1 -> Switch3/2
  Subsystem/1(Qref_hvdc_wfside) -> Wind farm side control/4(Qref_windfarm)
  Voltage Measurement5/1 -> V_DC Measure/1(In1)
  connections:
  Three-Phase V-I Measurement4/RConn3 -- 525 kV - 380 kV L = 0.1947 pu/LConn3
  Three-Phase V-I Measurement5/RConn1 -- Three-Level Bridge2/LConn1
  C/RConn1 -- Three-Phase V-I Measurement1/LConn3
  Lp3/LConn1 -- Series RLC Branch4/RConn1 -- Three-Level Bridge3/RConn3
  Series RLC Branch5/LConn1 -- V_DC_neg Measure/LConn1 -- Voltage Measurement5/LConn2 -- Three-Level Bridge2/RConn2 -- Series RLC Branch6/RConn1 -- Ground1/LConn1
  Smoothing Reactor/LConn1 -- DC Capacitor/LConn1 -- Three-Level Bridge3/RConn1
  Three-Phase V-I Measurement5/RConn3 -- Three-Level Bridge2/LConn3
  Series RLC Branch4/LConn1 -- DC Capacitor/RConn1 -- Three-Level Bridge3/RConn2 -- Ground2/LConn1
  Three-Phase V-I Measurement5/RConn2 -- Three-Level Bridge2/LConn2
  Three-Phase V-I Measurement1/RConn3 -- 220 kV - 525 kV L = 0.1947 pu/LConn3
  AC Filter wfside/LConn2 -- 220 kV - 525 kV L = 0.1947 pu/RConn2 -- Phase Reactor wfside L = 0.0781 pu/RConn2
  AC Filter gride side/LConn1 -- 525 kV - 380 kV L = 0.1947 pu/RConn1 -- Phase Reactor grid L = 0.0781 pu/LConn1
  Three-Phase V-I Measurement7/RConn3 -- Three-Level Bridge3/LConn3
  Three-Phase V-I Measurement4/LConn2 -- b/RConn1
  B/RConn1 -- Three-Phase V-I Measurement1/LConn2
  AC Filter gride side/LConn2 -- 525 kV - 380 kV L = 0.1947 pu/RConn2 -- Phase Reactor grid L = 0.0781 pu/LConn2
  Phase Reactor grid L = 0.0781 pu/RConn3 -- Three-Phase V-I Measurement5/LConn3
  Three-Phase V-I Measurement4/RConn1 -- 525 kV - 380 kV L = 0.1947 pu/LConn1
  a/RConn1 -- Three-Phase V-I Measurement4/LConn1
  Series RLC Branch6/LConn1 -- Voltage Measurement5/LConn1 -- Three-Level Bridge2/RConn1 -- Lp2/RConn1
  Lp2/LConn1 -- Current Measurement1/RConn1
  Three-Phase V-I Measurement4/LConn3 -- c/RConn1
  Ground6/LConn1 -- AC Filter gride side/RConn3 -- AC Filter gride side/RConn2 -- AC Filter gride side/RConn1
  AC Filter gride side/LConn3 -- 525 kV - 380 kV L = 0.1947 pu/RConn3 -- Phase Reactor grid L = 0.0781 pu/LConn3
  AC Filter wfside/RConn3 -- AC Filter wfside/RConn1 -- Ground4/LConn1 -- AC Filter wfside/RConn2
  Smoothing Reactor/RConn1 -- 45-km cable pos dc/LConn1
  45-km cable pos dc/RConn1 -- Current Measurement1/LConn1
  Current Measurement2/RConn1 -- 45-km cable neg dc/LConn1
  Three-Phase V-I Measurement7/RConn1 -- Three-Level Bridge3/LConn1
  AC Filter wfside/LConn1 -- 220 kV - 525 kV L = 0.1947 pu/RConn1 -- Phase Reactor wfside L = 0.0781 pu/RConn1
  Phase Reactor grid L = 0.0781 pu/RConn1 -- Three-Phase V-I Measurement5/LConn1
  A/RConn1 -- Three-Phase V-I Measurement1/LConn1
  Three-Phase V-I Measurement7/RConn2 -- Three-Level Bridge3/LConn2
  AC Filter wfside/LConn3 -- 220 kV - 525 kV L = 0.1947 pu/RConn3 -- Phase Reactor wfside L = 0.0781 pu/RConn3
  Three-Phase V-I Measurement7/LConn2 -- Phase Reactor wfside L = 0.0781 pu/LConn2
  Three-Phase V-I Measurement7/LConn1 -- Phase Reactor wfside L = 0.0781 pu/LConn1
  Lp4/LConn1 -- 45-km cable neg dc/RConn1
  Three-Phase V-I Measurement7/LConn3 -- Phase Reactor wfside L = 0.0781 pu/LConn3
  Three-Phase V-I Measurement4/RConn2 -- 525 kV - 380 kV L = 0.1947 pu/LConn2
  Three-Phase V-I Measurement1/RConn2 -- 220 kV - 525 kV L = 0.1947 pu/LConn2
  Three-Phase V-I Measurement1/RConn1 -- 220 kV - 525 kV L = 0.1947 pu/LConn1
  Three-Phase V-I Measurement5/LConn2 -- Phase Reactor grid L = 0.0781 pu/RConn2
  Lp4/RConn1 -- Series RLC Branch5/RConn1 -- Three-Level Bridge2/RConn3 -- V_DC_neg Measure/LConn2
  Lp3/RConn1 -- Current Measurement2/LConn1

== system Complete_Model/Power Computation ==
  Pout : spsSecondOrderFilterLib/Second-Order Filter  FilterType=Lowpass  Fo=17  Zeta=0.7  Ts=7.4074e-05  Initialize=off  Vac_Init="[0,  0,  60]"  Vdc_Init=0  PlotResponse=off  FreqRange=[0, 500, 1]
      settings: AttributesFormatString=f0=%<Fo>
  "Power (3ph, Instantaneous)7" : spsPower3phInstantaneousLib/Power (3ph, Instantaneous)
  Scope{20..23} (4 alike) : Scope
  Second-Order 1 : spsSecondOrderFilterLib/Second-Order Filter  FilterType=Lowpass  Fo=17  Zeta=0.7  Ts=7.4074e-05  Initialize=off  Vac_Init="[0,  0,  60]"  Vdc_Init=0  PlotResponse=off  FreqRange=[0, 500, 1]
      settings: AttributesFormatString=f0=%<Fo>
  To Workspace : ToWorkspace  VariableName=Vwf_tot  SaveFormat=Structure With Time  SampleTime=-1
  To Workspace1 : ToWorkspace  VariableName=Iwf_tot  SaveFormat=Structure With Time  SampleTime=-1
  To Workspace2 : ToWorkspace  VariableName=Pwind_tot  SaveFormat=Timeseries  SampleTime=-1
  To Workspace3 : ToWorkspace  VariableName=Qwind_tot  SaveFormat=Timeseries  SampleTime=-1
  ToFile_Iwf_tot : ToFile  Filename=Iwf_tot.mat  SaveFormat=Timeseries
  ToFile_Pwind_tot : ToFile  Filename=Pwind_tot.mat  SaveFormat=Timeseries
  ToFile_Qwind_tot : ToFile  Filename=Qwind_tot.mat  SaveFormat=Timeseries
  ToFile_Vwf_tot : ToFile  Filename=Vwf_tot.mat  SaveFormat=Timeseries
  tag scope: Pwind_tot=global, Qwind_tot=global
  wires:
  [Vwf_tot] -> ToFile_Vwf_tot/1, "Power (3ph, Instantaneous)7"/1, Scope21/1, To Workspace/1  "Vwf_tot"
  [Iwf_tot] -> ToFile_Iwf_tot/1, "Power (3ph, Instantaneous)7"/2, Scope20/1, To Workspace1/1  "Iwf_tot"
  "Power (3ph, Instantaneous)7"/1 -> Pout/1
  "Power (3ph, Instantaneous)7"/2 -> Second-Order 1/1
  Pout/1 -> ToFile_Pwind_tot/1, Scope22/1, To Workspace2/1, [Pwind_tot]  "Pwind_tot"
  Second-Order 1/1 -> ToFile_Qwind_tot/1, Scope23/1, To Workspace3/1, [Qwind_tot]  "Qwind_tot"

== system Complete_Model/Power Computation1 ==
  Pout : spsSecondOrderFilterLib/Second-Order Filter  FilterType=Lowpass  Fo=17  Zeta=0.7  Ts=7.4074e-05  Initialize=off  Vac_Init="[0,  0,  60]"  Vdc_Init=0  PlotResponse=off  FreqRange=[0, 500, 1]
      settings: AttributesFormatString=f0=%<Fo>
  Pout1 : spsSecondOrderFilterLib/Second-Order Filter  FilterType=Lowpass  Fo=17  Zeta=0.7  Ts=7.4074e-05  Initialize=off  Vac_Init="[0,  0,  60]"  Vdc_Init=0  PlotResponse=off  FreqRange=[0, 500, 1]
      settings: AttributesFormatString=f0=%<Fo>
  "Power (3ph, Instantaneous)7" : spsPower3phInstantaneousLib/Power (3ph, Instantaneous)
  Scope{1,20..23} (5 alike) : Scope
  To Workspace : ToWorkspace  VariableName=V_BE_grid_tot  SaveFormat=Structure With Time  SampleTime=-1
  To Workspace1 : ToWorkspace  VariableName=I_BE_grid_tot1  SaveFormat=Structure With Time  SampleTime=-1
  To Workspace2 : ToWorkspace  VariableName=P_BE_grid_tot  SaveFormat=Timeseries  SampleTime=-1
  To Workspace3 : ToWorkspace  VariableName=Q_BE_grid_tot  SaveFormat=Timeseries  SampleTime=-1
  ToFile_I_BE_grid_tot1 : ToFile  Filename=I_BE_grid_tot1.mat  SaveFormat=Timeseries
  ToFile_P_BE_grid_tot : ToFile  Filename=P_BE_grid_tot.mat  SaveFormat=Timeseries
  ToFile_Q_BE_grid_tot : ToFile  Filename=Q_BE_grid_tot.mat  SaveFormat=Timeseries
  ToFile_V_BE_grid_tot : ToFile  Filename=V_BE_grid_tot.mat  SaveFormat=Timeseries
  wires:
  "Power (3ph, Instantaneous)7"/2 -> Pout1/1
  "Power (3ph, Instantaneous)7"/1 -> Scope1/1, Pout/1
  [I_BE_grid_tot] -> To Workspace1/1, "Power (3ph, Instantaneous)7"/2, Scope20/1, ToFile_I_BE_grid_tot1/1  "I_BE_grid_tot1"
  [V_BE_grid_tot] -> To Workspace/1, "Power (3ph, Instantaneous)7"/1, Scope21/1, ToFile_V_BE_grid_tot/1  "V_BE_grid_tot"
  Pout/1 -> To Workspace2/1, Scope22/1, ToFile_P_BE_grid_tot/1  "P_BE_grid_tot"
  Pout1/1 -> To Workspace3/1, Scope23/1, ToFile_Q_BE_grid_tot/1  "Q_BE_grid_tot"

== system Complete_Model/ 3 AC Cables group 1/Power Computations ==
  Pout{,1..5} (6 alike) : spsSecondOrderFilterLib/Second-Order Filter  FilterType=Lowpass  Fo=17  Zeta=0.7  Ts=7.4074e-05  Initialize=off  Vac_Init="[0,  0,  60]"  Vdc_Init=0  PlotResponse=off  FreqRange=[0, 500, 1]
      settings: AttributesFormatString=f0=%<Fo>
  "Power (3ph, Instantaneous)5" : spsPower3phInstantaneousLib/Power (3ph, Instantaneous)
  "Power (3ph, Instantaneous)7" : spsPower3phInstantaneousLib/Power (3ph, Instantaneous)
  "Power (3ph, Instantaneous)8" : spsPower3phInstantaneousLib/Power (3ph, Instantaneous)
  Scope{14..17,19..24,26,29} (12 alike) : Scope
  To Workspace : ToWorkspace  VariableName=P_AC1_wind_filtered  SaveFormat=Timeseries  SampleTime=-1
  To Workspace1 : ToWorkspace  VariableName=P_AC1_wind  SaveFormat=Timeseries  SampleTime=-1
  To Workspace10 : ToWorkspace  VariableName=Q_AC1_grid  SaveFormat=Timeseries  SampleTime=-1
  To Workspace11 : ToWorkspace  VariableName=Q_AC1_grid_filtered  SaveFormat=Timeseries  SampleTime=-1
  To Workspace2 : ToWorkspace  VariableName=Q_AC1_wind  SaveFormat=Timeseries  SampleTime=-1
  To Workspace3 : ToWorkspace  VariableName=Q_AC1_wind_filtered  SaveFormat=Timeseries  SampleTime=-1
  To Workspace4 : ToWorkspace  VariableName=P_AC1_middle_filtered  SaveFormat=Timeseries  SampleTime=-1
  To Workspace5 : ToWorkspace  VariableName=P_AC1_middle  SaveFormat=Timeseries  SampleTime=-1
  To Workspace6 : ToWorkspace  VariableName=Q_AC1_middle_filtered  SaveFormat=Timeseries  SampleTime=-1
  To Workspace7 : ToWorkspace  VariableName=Q_AC1_middle  SaveFormat=Timeseries  SampleTime=-1
  To Workspace8 : ToWorkspace  VariableName=P_AC1_grid_filtered  SaveFormat=Timeseries  SampleTime=-1
  To Workspace9 : ToWorkspace  VariableName=P_AC1_grid  SaveFormat=Timeseries  SampleTime=-1
  ToFile_P_AC1_grid : ToFile  Filename=P_AC1_grid.mat  SaveFormat=Timeseries
  ToFile_P_AC1_grid_filtered : ToFile  Filename=P_AC1_grid_filtered.mat  SaveFormat=Timeseries
  ToFile_P_AC1_middle : ToFile  Filename=P_AC1_middle.mat  SaveFormat=Timeseries
  ToFile_P_AC1_middle_filtered : ToFile  Filename=P_AC1_middle_filtered.mat  SaveFormat=Timeseries
  ToFile_P_AC1_wind : ToFile  Filename=P_AC1_wind.mat  SaveFormat=Timeseries
  ToFile_P_AC1_wind_filtered : ToFile  SaveFormat=Timeseries
  ToFile_Q_AC1_grid : ToFile  Filename=Q_AC1_grid.mat  SaveFormat=Timeseries
  ToFile_Q_AC1_grid_filtered : ToFile  Filename=Q_AC1_grid_filtered.mat  SaveFormat=Timeseries
  ToFile_Q_AC1_middle : ToFile  Filename=Q_AC1_middle.mat  SaveFormat=Timeseries
  ToFile_Q_AC1_middle_filtered : ToFile  Filename=Q_AC1_middle_filtered.mat  SaveFormat=Timeseries
  ToFile_Q_AC1_wind : ToFile  Filename=Q_AC1_wind.mat  SaveFormat=Timeseries
  ToFile_Q_AC1_wind_filtered : ToFile  Filename=Q_AC1_wind_filtered.mat  SaveFormat=Timeseries
  wires:
  [Vcontinent220] -> Scope15/1, "Power (3ph, Instantaneous)8"/1
  "Power (3ph, Instantaneous)8"/2 -> Pout3/1, To Workspace7/1, ToFile_Q_AC1_middle/1  "Q_AC1_middle"
  "Power (3ph, Instantaneous)5"/1 -> To Workspace9/1, Pout5/1, ToFile_P_AC1_grid/1  "P_AC1_grid"
  [Ien_isl] -> Scope20/1, "Power (3ph, Instantaneous)7"/2
  "Power (3ph, Instantaneous)8"/1 -> To Workspace5/1, Pout2/1, ToFile_P_AC1_middle/1  "P_AC1_middle"
  [Icontinent] -> "Power (3ph, Instantaneous)5"/2, Scope24/1
  [Icontinent220] -> Scope14/1, "Power (3ph, Instantaneous)8"/2
  [Vcontinent] -> Scope26/1, "Power (3ph, Instantaneous)5"/1
  "Power (3ph, Instantaneous)7"/2 -> To Workspace2/1, Pout1/1, ToFile_Q_AC1_wind/1  "Q_AC1_wind"
  "Power (3ph, Instantaneous)7"/1 -> Pout/1, To Workspace1/1, ToFile_P_AC1_wind/1  "P_AC1_wind"
  "Power (3ph, Instantaneous)5"/2 -> To Workspace10/1, Pout4/1, ToFile_Q_AC1_grid/1  "Q_AC1_grid"
  [Ven_isl] -> Scope21/1, "Power (3ph, Instantaneous)7"/1
  Pout/1 -> To Workspace/1, Scope22/1  "Pwindfarm tot"
  Pout1/1 -> To Workspace3/1, Scope23/1, ToFile_Q_AC1_wind_filtered/1  "Q_AC1_wind_filtered"
  Pout2/1 -> To Workspace4/1, Scope16/1, ToFile_P_AC1_middle_filtered/1  "P_AC1_middle_filtered"
  Pout3/1 -> To Workspace6/1, Scope17/1, ToFile_Q_AC1_middle_filtered/1  "Q_AC1_middle_filtered"
  Pout4/1 -> To Workspace11/1, Scope19/1, ToFile_Q_AC1_grid_filtered/1  "Q_AC1_grid_filtered"
  Pout5/1 -> To Workspace8/1, Scope29/1, ToFile_P_AC1_grid_filtered/1  "P_AC1_grid_filtered"

== system Complete_Model/3 AC cables group 2/Power Computations ==
  Pout{1..6} (6 alike) : spsSecondOrderFilterLib/Second-Order Filter  FilterType=Lowpass  Fo=17  Zeta=0.7  Ts=7.4074e-05  Initialize=off  Vac_Init="[0,  0,  60]"  Vdc_Init=0  PlotResponse=off  FreqRange=[0, 500, 1]
      settings: AttributesFormatString=f0=%<Fo>
  "Power (3ph, Instantaneous)5" : spsPower3phInstantaneousLib/Power (3ph, Instantaneous)
  "Power (3ph, Instantaneous)7" : spsPower3phInstantaneousLib/Power (3ph, Instantaneous)
  "Power (3ph, Instantaneous)8" : spsPower3phInstantaneousLib/Power (3ph, Instantaneous)
  Scope{14..17,19..24,26,29} (12 alike) : Scope
  To Workspace : ToWorkspace  VariableName=P_AC2_wind_filtered  SaveFormat=Timeseries  SampleTime=-1
  To Workspace1 : ToWorkspace  VariableName=P_AC2_wind  SaveFormat=Timeseries  SampleTime=-1
  To Workspace10 : ToWorkspace  VariableName=Q_AC2_grid  SaveFormat=Timeseries  SampleTime=-1
  To Workspace11 : ToWorkspace  VariableName=Q_AC2_grid_filtered  SaveFormat=Timeseries  SampleTime=-1
  To Workspace12 : ToWorkspace  VariableName=Vsh_grid2  SaveFormat=Timeseries  SampleTime=-1
  To Workspace13 : ToWorkspace  VariableName=Ish_grid2  SaveFormat=Timeseries  SampleTime=-1
  To Workspace14 : ToWorkspace  VariableName=Vsh_wind2  SaveFormat=Timeseries  SampleTime=-1
  To Workspace15 : ToWorkspace  VariableName=Ish_wind2  SaveFormat=Timeseries  SampleTime=-1
  To Workspace2 : ToWorkspace  VariableName=Q_AC2_wind  SaveFormat=Timeseries  SampleTime=-1
  To Workspace3 : ToWorkspace  VariableName=Q_AC2_wind_filtered  SaveFormat=Timeseries  SampleTime=-1
  To Workspace4 : ToWorkspace  VariableName=P_AC2_middle_filtered  SaveFormat=Timeseries  SampleTime=-1
  To Workspace5 : ToWorkspace  VariableName=P_AC2_middle  SaveFormat=Timeseries  SampleTime=-1
  To Workspace6 : ToWorkspace  VariableName=Q_AC2_middle_filtered  SaveFormat=Timeseries  SampleTime=-1
  To Workspace7 : ToWorkspace  VariableName=Q_AC2_middle  SaveFormat=Timeseries  SampleTime=-1
  To Workspace8 : ToWorkspace  VariableName=P_AC2_grid_filtered  SaveFormat=Timeseries  SampleTime=-1
  To Workspace9 : ToWorkspace  VariableName=P_AC2_grid  SaveFormat=Timeseries  SampleTime=-1
  ToFile_Ish_grid2 : ToFile  Filename=Ish_grid2.mat  SaveFormat=Timeseries
  ToFile_Ish_wind2 : ToFile  Filename=Ish_wind2.mat  SaveFormat=Timeseries
  ToFile_P_AC2_grid : ToFile  Filename=P_AC2_grid.mat  SaveFormat=Timeseries
  ToFile_P_AC2_grid_filtered : ToFile  Filename=P_AC2_grid_filtered.mat  SaveFormat=Timeseries
  ToFile_P_AC2_middle : ToFile  Filename=P_AC2_middle.mat  SaveFormat=Timeseries
  ToFile_P_AC2_middle_filtered : ToFile  Filename=P_AC2_middle_filtered.mat  SaveFormat=Timeseries
  ToFile_P_AC2_wind : ToFile  Filename=P_AC2_wind.mat  SaveFormat=Timeseries
  ToFile_P_AC2_wind_filtered : ToFile  Filename=P_AC2_wind_filtered.mat  SaveFormat=Timeseries
  ToFile_Q_AC2_grid : ToFile  Filename=Q_AC2_grid.mat  SaveFormat=Timeseries
  ToFile_Q_AC2_grid_filtered : ToFile  Filename=Q_AC2_grid_filtered.mat  SaveFormat=Timeseries
  ToFile_Q_AC2_middle : ToFile  Filename=Q_AC2_middle.mat  SaveFormat=Timeseries
  ToFile_Q_AC2_middle_filtered : ToFile  Filename=Q_AC2_middle_filtered.mat  SaveFormat=Timeseries
  ToFile_Q_AC2_wind : ToFile  Filename=Q_AC2_wind.mat  SaveFormat=Timeseries
  ToFile_Q_AC2_wind_filtered : ToFile  Filename=Q_AC2_wind_filtered.mat  SaveFormat=Timeseries
  ToFile_Vsh_grid2 : ToFile  Filename=Vsh_grid2.mat  SaveFormat=Timeseries
  ToFile_Vsh_wind2 : ToFile  Filename=Vsh_wind2.mat  SaveFormat=Timeseries
  wires:
  "Power (3ph, Instantaneous)8"/2 -> To Workspace7/1, Pout3/1, ToFile_Q_AC2_middle/1  "Q_AC2_middle"
  [Icontinent220_2] -> Scope14/1, "Power (3ph, Instantaneous)8"/2
  "Power (3ph, Instantaneous)8"/1 -> ToFile_P_AC2_middle/1, Pout2/1, To Workspace5/1  "P_AC2_middle"
  [Vcontinent2] -> Scope26/1, "Power (3ph, Instantaneous)5"/1
  "Power (3ph, Instantaneous)5"/1 -> ToFile_P_AC2_grid/1, Pout4/1, To Workspace9/1  "P_AC2_grid"
  Pout4/1 -> ToFile_P_AC2_grid_filtered/1, Scope29/1, To Workspace8/1  "P_AC2_grid_filtered"
  [Ien_isl2] -> "Power (3ph, Instantaneous)7"/2, Scope20/1
  [Ish_wind2] -> To Workspace15/1, ToFile_Ish_wind2/1  "Ish_wind2"
  "Power (3ph, Instantaneous)5"/2 -> To Workspace10/1, Pout6/1, ToFile_Q_AC2_grid/1  "Q_AC2_grid"
  [Ven_isl2] -> "Power (3ph, Instantaneous)7"/1, Scope21/1
  [Vsh_grid2] -> To Workspace12/1, ToFile_Vsh_grid2/1  "Vsh_grid2"
  [Vsh_wind2] -> To Workspace14/1, ToFile_Vsh_wind2/1  "Vsh_wind2"
  [Ish_grid2] -> To Workspace13/1, ToFile_Ish_grid2/1  "Ish_grid2"
  "Power (3ph, Instantaneous)7"/1 -> To Workspace1/1, Pout5/1, ToFile_P_AC2_wind/1  "P_AC2_wind"
  Pout5/1 -> ToFile_P_AC2_wind_filtered/1, Scope22/1, To Workspace/1  "P_AC2_wind_filtered"
  [Icontinent2] -> "Power (3ph, Instantaneous)5"/2, Scope24/1
  "Power (3ph, Instantaneous)7"/2 -> ToFile_Q_AC2_wind/1, Pout1/1, To Workspace2/1  "Q_AC2_wind"
  [Vcontinent220_2] -> Scope15/1, "Power (3ph, Instantaneous)8"/1
  Pout3/1 -> ToFile_Q_AC2_middle_filtered/1, Scope17/1, To Workspace6/1  "Q_AC2_middle_filtered"
  Pout6/1 -> ToFile_Q_AC2_grid_filtered/1, Scope19/1, To Workspace11/1  "Q_AC2_grid_filtered"
  Pout1/1 -> ToFile_Q_AC2_wind_filtered/1, To Workspace3/1, Scope23/1  "Q_AC2_wind_filtered"
  Pout2/1 -> ToFile_P_AC2_middle_filtered/1, Scope16/1, To Workspace4/1  "P_AC2_middle_filtered"
